simulink model "pfb_fft_pow_ab_16x"  (R2013a) (continued)
== system pfb_fft_pow_ab_16x == (continued)
  pipeline5 : Subsystem  AncestorBlock=casper_library_delays/pipeline  mask(pipeline){latency=1}
      mask help:
      | An explicitly laid-out delay line to avoid mapping to SRL16s
      | for use in pipelining to help achieve timing closure.
      mask init:
      | pipeline_init(gcb,'latency',latency);
      settings: AttributesFormatString=Latency = %<latency>
      mask Display:
      | port_label('input', 1, 'd');
      | port_label('output', 1, 'q');
      | disp(['Z^-', num2str(latency)]);
  pipeline50 : Subsystem  AncestorBlock=casper_library_delays/pipeline  mask(pipeline){latency=1}
      mask help:
      | An explicitly laid-out delay line to avoid mapping to SRL16s
      | for use in pipelining to help achieve timing closure.
      mask init:
      | pipeline_init(gcb,'latency',latency);
      settings: AttributesFormatString=Latency = %<latency>
      mask Display:
      | port_label('input', 1, 'd');
      | port_label('output', 1, 'q');
      | disp(['Z^-', num2str(latency)]);
  pipeline51 : Subsystem  AncestorBlock=casper_library_delays/pipeline  mask(pipeline){latency=1}
      mask help:
      | An explicitly laid-out delay line to avoid mapping to SRL16s
      | for use in pipelining to help achieve timing closure.
      mask init:
      | pipeline_init(gcb,'latency',latency);
      settings: AttributesFormatString=Latency = %<latency>
      mask Display:
      | port_label('input', 1, 'd');
      | port_label('output', 1, 'q');
      | disp(['Z^-', num2str(latency)]);
  pipeline52 : Subsystem  AncestorBlock=casper_library_delays/pipeline  mask(pipeline){latency=2}
      mask help:
      | An explicitly laid-out delay line to avoid mapping to SRL16s
      | for use in pipelining to help achieve timing closure.
      mask init:
      | pipeline_init(gcb,'latency',latency);
      settings: AttributesFormatString=Latency = %<latency>
      mask Display:
      | port_label('input', 1, 'd');
      | port_label('output', 1, 'q');
      | disp(['Z^-', num2str(latency)]);
  pipeline53 : Subsystem  AncestorBlock=casper_library_delays/pipeline  mask(pipeline){latency=1}
      mask help:
      | An explicitly laid-out delay line to avoid mapping to SRL16s
      | for use in pipelining to help achieve timing closure.
      mask init:
      | pipeline_init(gcb,'latency',latency);
      settings: AttributesFormatString=Latency = %<latency>
      mask Display:
      | port_label('input', 1, 'd');
      | port_label('output', 1, 'q');
      | disp(['Z^-', num2str(latency)]);
  pipeline54 : Subsystem  AncestorBlock=casper_library_delays/pipeline  mask(pipeline){latency=2}
      mask help:
      | An explicitly laid-out delay line to avoid mapping to SRL16s
      | for use in pipelining to help achieve timing closure.
      mask init:
      | pipeline_init(gcb,'latency',latency);
      settings: AttributesFormatString=Latency = %<latency>
      mask Display:
      | port_label('input', 1, 'd');
      | port_label('output', 1, 'q');
      | disp(['Z^-', num2str(latency)]);
  pipeline55 : Subsystem  AncestorBlock=casper_library_delays/pipeline  mask(pipeline){latency=2}
      mask help:
      | An explicitly laid-out delay line to avoid mapping to SRL16s
      | for use in pipelining to help achieve timing closure.
      mask init:
      | pipeline_init(gcb,'latency',latency);
      settings: AttributesFormatString=Latency = %<latency>
      mask Display:
      | port_label('input', 1, 'd');
      | port_label('output', 1, 'q');
      | disp(['Z^-', num2str(latency)]);
  pipeline56 : Subsystem  AncestorBlock=casper_library_delays/pipeline  mask(pipeline){latency=2}
      mask help:
      | An explicitly laid-out delay line to avoid mapping to SRL16s
      | for use in pipelining to help achieve timing closure.
      mask init:
      | pipeline_init(gcb,'latency',latency);
      settings: AttributesFormatString=Latency = %<latency>
      mask Display:
      | port_label('input', 1, 'd');
      | port_label('output', 1, 'q');
      | disp(['Z^-', num2str(latency)]);
  pipeline57 : Subsystem  AncestorBlock=casper_library_delays/pipeline  mask(pipeline){latency=2}
      mask help:
      | An explicitly laid-out delay line to avoid mapping to SRL16s
      | for use in pipelining to help achieve timing closure.
      mask init:
      | pipeline_init(gcb,'latency',latency);
      settings: AttributesFormatString=Latency = %<latency>
      mask Display:
      | port_label('input', 1, 'd');
      | port_label('output', 1, 'q');
      | disp(['Z^-', num2str(latency)]);
  pipeline58 : Subsystem  AncestorBlock=casper_library_delays/pipeline  mask(pipeline){latency=1}
      mask help:
      | An explicitly laid-out delay line to avoid mapping to SRL16s
      | for use in pipelining to help achieve timing closure.
      mask init:
      | pipeline_init(gcb,'latency',latency);
      settings: AttributesFormatString=Latency = %<latency>
      mask Display:
      | port_label('input', 1, 'd');
      | port_label('output', 1, 'q');
      | disp(['Z^-', num2str(latency)]);
  pipeline59 : Subsystem  AncestorBlock=casper_library_delays/pipeline  mask(pipeline){latency=1}
      mask help:
      | An explicitly laid-out delay line to avoid mapping to SRL16s
      | for use in pipelining to help achieve timing closure.
      mask init:
      | pipeline_init(gcb,'latency',latency);
      settings: AttributesFormatString=Latency = %<latency>
      mask Display:
      | port_label('input', 1, 'd');
      | port_label('output', 1, 'q');
      | disp(['Z^-', num2str(latency)]);
  pipeline6 : Subsystem  AncestorBlock=casper_library_delays/pipeline  mask(pipeline){latency=1}
      mask help:
      | An explicitly laid-out delay line to avoid mapping to SRL16s
      | for use in pipelining to help achieve timing closure.
      mask init:
      | pipeline_init(gcb,'latency',latency);
      settings: AttributesFormatString=Latency = %<latency>
      mask Display:
      | port_label('input', 1, 'd');
      | port_label('output', 1, 'q');
      | disp(['Z^-', num2str(latency)]);
  pipeline60 : Subsystem  AncestorBlock=casper_library_delays/pipeline  mask(pipeline){latency=1}
      mask help:
      | An explicitly laid-out delay line to avoid mapping to SRL16s
      | for use in pipelining to help achieve timing closure.
      mask init:
      | pipeline_init(gcb,'latency',latency);
      settings: AttributesFormatString=Latency = %<latency>
      mask Display:
      | port_label('input', 1, 'd');
      | port_label('output', 1, 'q');
      | disp(['Z^-', num2str(latency)]);
  pipeline61 : Subsystem  AncestorBlock=casper_library_delays/pipeline  mask(pipeline){latency=1}
      mask help:
      | An explicitly laid-out delay line to avoid mapping to SRL16s
      | for use in pipelining to help achieve timing closure.
      mask init:
      | pipeline_init(gcb,'latency',latency);
      settings: AttributesFormatString=Latency = %<latency>
      mask Display:
      | port_label('input', 1, 'd');
      | port_label('output', 1, 'q');
      | disp(['Z^-', num2str(latency)]);
  pipeline62 : Subsystem  AncestorBlock=casper_library_delays/pipeline  mask(pipeline){latency=1}
      mask help:
      | An explicitly laid-out delay line to avoid mapping to SRL16s
      | for use in pipelining to help achieve timing closure.
      mask init:
      | pipeline_init(gcb,'latency',latency);
      settings: AttributesFormatString=Latency = %<latency>
      mask Display:
      | port_label('input', 1, 'd');
      | port_label('output', 1, 'q');
      | disp(['Z^-', num2str(latency)]);
  pipeline63 : Subsystem  AncestorBlock=casper_library_delays/pipeline  mask(pipeline){latency=1}
      mask help:
      | An explicitly laid-out delay line to avoid mapping to SRL16s
      | for use in pipelining to help achieve timing closure.
      mask init:
      | pipeline_init(gcb,'latency',latency);
      settings: AttributesFormatString=Latency = %<latency>
      mask Display:
      | port_label('input', 1, 'd');
      | port_label('output', 1, 'q');
      | disp(['Z^-', num2str(latency)]);
  pipeline64 : Subsystem  AncestorBlock=casper_library_delays/pipeline  mask(pipeline){latency=1}
      mask help:
      | An explicitly laid-out delay line to avoid mapping to SRL16s
      | for use in pipelining to help achieve timing closure.
      mask init:
      | pipeline_init(gcb,'latency',latency);
      settings: AttributesFormatString=Latency = %<latency>
      mask Display:
      | port_label('input', 1, 'd');
      | port_label('output', 1, 'q');
      | disp(['Z^-', num2str(latency)]);
  pipeline65 : Subsystem  AncestorBlock=casper_library_delays/pipeline  mask(pipeline){latency=1}
      mask help:
      | An explicitly laid-out delay line to avoid mapping to SRL16s
      | for use in pipelining to help achieve timing closure.
      mask init:
      | pipeline_init(gcb,'latency',latency);
      settings: AttributesFormatString=Latency = %<latency>
      mask Display:
      | port_label('input', 1, 'd');
      | port_label('output', 1, 'q');
      | disp(['Z^-', num2str(latency)]);
  pipeline66 : Subsystem  AncestorBlock=casper_library_delays/pipeline  mask(pipeline){latency=1}
      mask help:
      | An explicitly laid-out delay line to avoid mapping to SRL16s
      | for use in pipelining to help achieve timing closure.
      mask init:
      | pipeline_init(gcb,'latency',latency);
      settings: AttributesFormatString=Latency = %<latency>
      mask Display:
      | port_label('input', 1, 'd');
      | port_label('output', 1, 'q');
      | disp(['Z^-', num2str(latency)]);
  pipeline67 : Subsystem  AncestorBlock=casper_library_delays/pipeline  mask(pipeline){latency=1}
      mask help:
      | An explicitly laid-out delay line to avoid mapping to SRL16s
      | for use in pipelining to help achieve timing closure.
      mask init:
      | pipeline_init(gcb,'latency',latency);
      settings: AttributesFormatString=Latency = %<latency>
      mask Display:
      | port_label('input', 1, 'd');
      | port_label('output', 1, 'q');
      | disp(['Z^-', num2str(latency)]);
  pipeline68 : Subsystem  AncestorBlock=casper_library_delays/pipeline  mask(pipeline){latency=1}
      mask help:
      | An explicitly laid-out delay line to avoid mapping to SRL16s
      | for use in pipelining to help achieve timing closure.
      mask init:
      | pipeline_init(gcb,'latency',latency);
      settings: AttributesFormatString=Latency = %<latency>
      mask Display:
      | port_label('input', 1, 'd');
      | port_label('output', 1, 'q');
      | disp(['Z^-', num2str(latency)]);
  pipeline69 : Subsystem  AncestorBlock=casper_library_delays/pipeline  mask(pipeline){latency=1}
      mask help:
      | An explicitly laid-out delay line to avoid mapping to SRL16s
      | for use in pipelining to help achieve timing closure.
      mask init:
      | pipeline_init(gcb,'latency',latency);
      settings: AttributesFormatString=Latency = %<latency>
      mask Display:
      | port_label('input', 1, 'd');
      | port_label('output', 1, 'q');
      | disp(['Z^-', num2str(latency)]);
  pipeline7 : Subsystem  AncestorBlock=casper_library_delays/pipeline  mask(pipeline){latency=1}
      mask help:
      | An explicitly laid-out delay line to avoid mapping to SRL16s
      | for use in pipelining to help achieve timing closure.
      mask init:
      | pipeline_init(gcb,'latency',latency);
      settings: AttributesFormatString=Latency = %<latency>
      mask Display:
      | port_label('input', 1, 'd');
      | port_label('output', 1, 'q');
      | disp(['Z^-', num2str(latency)]);
  pipeline70 : Subsystem  AncestorBlock=casper_library_delays/pipeline  mask(pipeline){latency=1}
      mask help:
      | An explicitly laid-out delay line to avoid mapping to SRL16s
      | for use in pipelining to help achieve timing closure.
      mask init:
      | pipeline_init(gcb,'latency',latency);
      settings: AttributesFormatString=Latency = %<latency>
      mask Display:
      | port_label('input', 1, 'd');
      | port_label('output', 1, 'q');
      | disp(['Z^-', num2str(latency)]);
  pipeline71 : Subsystem  AncestorBlock=casper_library_delays/pipeline  mask(pipeline){latency=1}
      mask help:
      | An explicitly laid-out delay line to avoid mapping to SRL16s
      | for use in pipelining to help achieve timing closure.
      mask init:
      | pipeline_init(gcb,'latency',latency);
      settings: AttributesFormatString=Latency = %<latency>
      mask Display:
      | port_label('input', 1, 'd');
      | port_label('output', 1, 'q');
      | disp(['Z^-', num2str(latency)]);
  pipeline72 : Subsystem  AncestorBlock=casper_library_delays/pipeline  mask(pipeline){latency=1}
      mask help:
      | An explicitly laid-out delay line to avoid mapping to SRL16s
      | for use in pipelining to help achieve timing closure.
      mask init:
      | pipeline_init(gcb,'latency',latency);
      settings: AttributesFormatString=Latency = %<latency>
      mask Display:
      | port_label('input', 1, 'd');
      | port_label('output', 1, 'q');
      | disp(['Z^-', num2str(latency)]);
  pipeline73 : Subsystem  AncestorBlock=casper_library_delays/pipeline  mask(pipeline){latency=1}
      mask help:
      | An explicitly laid-out delay line to avoid mapping to SRL16s
      | for use in pipelining to help achieve timing closure.
      mask init:
      | pipeline_init(gcb,'latency',latency);
      settings: AttributesFormatString=Latency = %<latency>
      mask Display:
      | port_label('input', 1, 'd');
      | port_label('output', 1, 'q');
      | disp(['Z^-', num2str(latency)]);
  pipeline74 : Subsystem  AncestorBlock=casper_library_delays/pipeline  mask(pipeline){latency=1}
      mask help:
      | An explicitly laid-out delay line to avoid mapping to SRL16s
      | for use in pipelining to help achieve timing closure.
      mask init:
      | pipeline_init(gcb,'latency',latency);
      settings: AttributesFormatString=Latency = %<latency>
      mask Display:
      | port_label('input', 1, 'd');
      | port_label('output', 1, 'q');
      | disp(['Z^-', num2str(latency)]);
  pipeline75 : Subsystem  AncestorBlock=casper_library_delays/pipeline  mask(pipeline){latency=1}
      mask help:
      | An explicitly laid-out delay line to avoid mapping to SRL16s
      | for use in pipelining to help achieve timing closure.
      mask init:
      | pipeline_init(gcb,'latency',latency);
      settings: AttributesFormatString=Latency = %<latency>
      mask Display:
      | port_label('input', 1, 'd');
      | port_label('output', 1, 'q');
      | disp(['Z^-', num2str(latency)]);
  pipeline76 : Subsystem  AncestorBlock=casper_library_delays/pipeline  mask(pipeline){latency=1}
      mask help:
      | An explicitly laid-out delay line to avoid mapping to SRL16s
      | for use in pipelining to help achieve timing closure.
      mask init:
      | pipeline_init(gcb,'latency',latency);
      settings: AttributesFormatString=Latency = %<latency>
      mask Display:
      | port_label('input', 1, 'd');
      | port_label('output', 1, 'q');
      | disp(['Z^-', num2str(latency)]);
  pipeline77 : Subsystem  AncestorBlock=casper_library_delays/pipeline  mask(pipeline){latency=1}
      mask help:
      | An explicitly laid-out delay line to avoid mapping to SRL16s
      | for use in pipelining to help achieve timing closure.
      mask init:
      | pipeline_init(gcb,'latency',latency);
      settings: AttributesFormatString=Latency = %<latency>
      mask Display:
      | port_label('input', 1, 'd');
      | port_label('output', 1, 'q');
      | disp(['Z^-', num2str(latency)]);
  pipeline78 : Subsystem  AncestorBlock=casper_library_delays/pipeline  mask(pipeline){latency=1}
      mask help:
      | An explicitly laid-out delay line to avoid mapping to SRL16s
      | for use in pipelining to help achieve timing closure.
      mask init:
      | pipeline_init(gcb,'latency',latency);
      settings: AttributesFormatString=Latency = %<latency>
      mask Display:
      | port_label('input', 1, 'd');
      | port_label('output', 1, 'q');
      | disp(['Z^-', num2str(latency)]);
  pipeline79 : Subsystem  AncestorBlock=casper_library_delays/pipeline  mask(pipeline){latency=1}
      mask help:
      | An explicitly laid-out delay line to avoid mapping to SRL16s
      | for use in pipelining to help achieve timing closure.
      mask init:
      | pipeline_init(gcb,'latency',latency);
      settings: AttributesFormatString=Latency = %<latency>
      mask Display:
      | port_label('input', 1, 'd');
      | port_label('output', 1, 'q');
      | disp(['Z^-', num2str(latency)]);
  pipeline8 : Subsystem  AncestorBlock=casper_library_delays/pipeline  mask(pipeline){latency=1}
      mask help:
      | An explicitly laid-out delay line to avoid mapping to SRL16s
      | for use in pipelining to help achieve timing closure.
      mask init:
      | pipeline_init(gcb,'latency',latency);
      settings: AttributesFormatString=Latency = %<latency>
      mask Display:
      | port_label('input', 1, 'd');
      | port_label('output', 1, 'q');
      | disp(['Z^-', num2str(latency)]);
  pipeline80 : Subsystem  AncestorBlock=casper_library_delays/pipeline  mask(pipeline){latency=1}
      mask help:
      | An explicitly laid-out delay line to avoid mapping to SRL16s
      | for use in pipelining to help achieve timing closure.
      mask init:
      | pipeline_init(gcb,'latency',latency);
      settings: AttributesFormatString=Latency = %<latency>
      mask Display:
      | port_label('input', 1, 'd');
      | port_label('output', 1, 'q');
      | disp(['Z^-', num2str(latency)]);
  pipeline81 : Subsystem  AncestorBlock=casper_library_delays/pipeline  mask(pipeline){latency=1}
      mask help:
      | An explicitly laid-out delay line to avoid mapping to SRL16s
      | for use in pipelining to help achieve timing closure.
      mask init:
      | pipeline_init(gcb,'latency',latency);
      settings: AttributesFormatString=Latency = %<latency>
      mask Display:
      | port_label('input', 1, 'd');
      | port_label('output', 1, 'q');
      | disp(['Z^-', num2str(latency)]);
  pipeline82 : Subsystem  AncestorBlock=casper_library_delays/pipeline  mask(pipeline){latency=1}
      mask help:
      | An explicitly laid-out delay line to avoid mapping to SRL16s
      | for use in pipelining to help achieve timing closure.
      mask init:
      | pipeline_init(gcb,'latency',latency);
      settings: AttributesFormatString=Latency = %<latency>
      mask Display:
      | port_label('input', 1, 'd');
      | port_label('output', 1, 'q');
      | disp(['Z^-', num2str(latency)]);
  pipeline83 : Subsystem  AncestorBlock=casper_library_delays/pipeline  mask(pipeline){latency=1}
      mask help:
      | An explicitly laid-out delay line to avoid mapping to SRL16s
      | for use in pipelining to help achieve timing closure.
      mask init:
      | pipeline_init(gcb,'latency',latency);
      settings: AttributesFormatString=Latency = %<latency>
      mask Display:
      | port_label('input', 1, 'd');
      | port_label('output', 1, 'q');
      | disp(['Z^-', num2str(latency)]);
  pipeline84 : Subsystem  AncestorBlock=casper_library_delays/pipeline  mask(pipeline){latency=2}
      mask help:
      | An explicitly laid-out delay line to avoid mapping to SRL16s
      | for use in pipelining to help achieve timing closure.
      mask init:
      | pipeline_init(gcb,'latency',latency);
      settings: AttributesFormatString=Latency = %<latency>
      mask Display:
      | port_label('input', 1, 'd');
      | port_label('output', 1, 'q');
      | disp(['Z^-', num2str(latency)]);
  pipeline85 : Subsystem  AncestorBlock=casper_library_delays/pipeline  mask(pipeline){latency=1}
      mask help:
      | An explicitly laid-out delay line to avoid mapping to SRL16s
      | for use in pipelining to help achieve timing closure.
      mask init:
      | pipeline_init(gcb,'latency',latency);
      settings: AttributesFormatString=Latency = %<latency>
      mask Display:
      | port_label('input', 1, 'd');
      | port_label('output', 1, 'q');
      | disp(['Z^-', num2str(latency)]);
  pipeline86 : Subsystem  AncestorBlock=casper_library_delays/pipeline  mask(pipeline){latency=2}
      mask help:
      | An explicitly laid-out delay line to avoid mapping to SRL16s
      | for use in pipelining to help achieve timing closure.
      mask init:
      | pipeline_init(gcb,'latency',latency);
      settings: AttributesFormatString=Latency = %<latency>
      mask Display:
      | port_label('input', 1, 'd');
      | port_label('output', 1, 'q');
      | disp(['Z^-', num2str(latency)]);
  pipeline87 : Subsystem  AncestorBlock=casper_library_delays/pipeline  mask(pipeline){latency=2}
      mask help:
      | An explicitly laid-out delay line to avoid mapping to SRL16s
      | for use in pipelining to help achieve timing closure.
      mask init:
      | pipeline_init(gcb,'latency',latency);
      settings: AttributesFormatString=Latency = %<latency>
      mask Display:
      | port_label('input', 1, 'd');
      | port_label('output', 1, 'q');
      | disp(['Z^-', num2str(latency)]);
  pipeline88 : Subsystem  AncestorBlock=casper_library_delays/pipeline  mask(pipeline){latency=2}
      mask help:
      | An explicitly laid-out delay line to avoid mapping to SRL16s
      | for use in pipelining to help achieve timing closure.
      mask init:
      | pipeline_init(gcb,'latency',latency);
      settings: AttributesFormatString=Latency = %<latency>
      mask Display:
      | port_label('input', 1, 'd');
      | port_label('output', 1, 'q');
      | disp(['Z^-', num2str(latency)]);
  pipeline89 : Subsystem  AncestorBlock=casper_library_delays/pipeline  mask(pipeline){latency=2}
      mask help:
      | An explicitly laid-out delay line to avoid mapping to SRL16s
      | for use in pipelining to help achieve timing closure.
      mask init:
      | pipeline_init(gcb,'latency',latency);
      settings: AttributesFormatString=Latency = %<latency>
      mask Display:
      | port_label('input', 1, 'd');
      | port_label('output', 1, 'q');
      | disp(['Z^-', num2str(latency)]);
  pipeline9 : Subsystem  AncestorBlock=casper_library_delays/pipeline  mask(pipeline){latency=1}
      mask help:
      | An explicitly laid-out delay line to avoid mapping to SRL16s
      | for use in pipelining to help achieve timing closure.
      mask init:
      | pipeline_init(gcb,'latency',latency);
      settings: AttributesFormatString=Latency = %<latency>
      mask Display:
      | port_label('input', 1, 'd');
      | port_label('output', 1, 'q');
      | disp(['Z^-', num2str(latency)]);
  pipeline90 : Subsystem  AncestorBlock=casper_library_delays/pipeline  mask(pipeline){latency=1}
      mask help:
      | An explicitly laid-out delay line to avoid mapping to SRL16s
      | for use in pipelining to help achieve timing closure.
      mask init:
      | pipeline_init(gcb,'latency',latency);
      settings: AttributesFormatString=Latency = %<latency>
      mask Display:
      | port_label('input', 1, 'd');
      | port_label('output', 1, 'q');
      | disp(['Z^-', num2str(latency)]);
  pipeline91 : Subsystem  AncestorBlock=casper_library_delays/pipeline  mask(pipeline){latency=1}
      mask help:
      | An explicitly laid-out delay line to avoid mapping to SRL16s
      | for use in pipelining to help achieve timing closure.
      mask init:
      | pipeline_init(gcb,'latency',latency);
      settings: AttributesFormatString=Latency = %<latency>
      mask Display:
      | port_label('input', 1, 'd');
      | port_label('output', 1, 'q');
      | disp(['Z^-', num2str(latency)]);
  pipeline92 : Subsystem  AncestorBlock=casper_library_delays/pipeline  mask(pipeline){latency=1}
      mask help:
      | An explicitly laid-out delay line to avoid mapping to SRL16s
      | for use in pipelining to help achieve timing closure.
      mask init:
      | pipeline_init(gcb,'latency',latency);
      settings: AttributesFormatString=Latency = %<latency>
      mask Display:
      | port_label('input', 1, 'd');
      | port_label('output', 1, 'q');
      | disp(['Z^-', num2str(latency)]);
  pipeline93 : Subsystem  AncestorBlock=casper_library_delays/pipeline  mask(pipeline){latency=1}
      mask help:
      | An explicitly laid-out delay line to avoid mapping to SRL16s
      | for use in pipelining to help achieve timing closure.
      mask init:
      | pipeline_init(gcb,'latency',latency);
      settings: AttributesFormatString=Latency = %<latency>
      mask Display:
      | port_label('input', 1, 'd');
      | port_label('output', 1, 'q');
      | disp(['Z^-', num2str(latency)]);
  pipeline94 : Subsystem  AncestorBlock=casper_library_delays/pipeline  mask(pipeline){latency=1}
      mask help:
      | An explicitly laid-out delay line to avoid mapping to SRL16s
      | for use in pipelining to help achieve timing closure.
      mask init:
      | pipeline_init(gcb,'latency',latency);
      settings: AttributesFormatString=Latency = %<latency>
      mask Display:
      | port_label('input', 1, 'd');
      | port_label('output', 1, 'q');
      | disp(['Z^-', num2str(latency)]);
  pipeline95 : Subsystem  AncestorBlock=casper_library_delays/pipeline  mask(pipeline){latency=1}
      mask help:
      | An explicitly laid-out delay line to avoid mapping to SRL16s
      | for use in pipelining to help achieve timing closure.
      mask init:
      | pipeline_init(gcb,'latency',latency);
      settings: AttributesFormatString=Latency = %<latency>
      mask Display:
      | port_label('input', 1, 'd');
      | port_label('output', 1, 'q');
      | disp(['Z^-', num2str(latency)]);
  pipeline96 : Subsystem  AncestorBlock=casper_library_delays/pipeline  mask(pipeline){latency=1}
      mask help:
      | An explicitly laid-out delay line to avoid mapping to SRL16s
      | for use in pipelining to help achieve timing closure.
      mask init:
      | pipeline_init(gcb,'latency',latency);
      settings: AttributesFormatString=Latency = %<latency>
      mask Display:
      | port_label('input', 1, 'd');
      | port_label('output', 1, 'q');
      | disp(['Z^-', num2str(latency)]);
  pipeline97 : Subsystem  AncestorBlock=casper_library_delays/pipeline  mask(pipeline){latency=1}
      mask help:
      | An explicitly laid-out delay line to avoid mapping to SRL16s
      | for use in pipelining to help achieve timing closure.
      mask init:
      | pipeline_init(gcb,'latency',latency);
      settings: AttributesFormatString=Latency = %<latency>
      mask Display:
      | port_label('input', 1, 'd');
      | port_label('output', 1, 'q');
      | disp(['Z^-', num2str(latency)]);
  pipeline98 : Subsystem  AncestorBlock=casper_library_delays/pipeline  mask(pipeline){latency=1}
      mask help:
      | An explicitly laid-out delay line to avoid mapping to SRL16s
      | for use in pipelining to help achieve timing closure.
      mask init:
      | pipeline_init(gcb,'latency',latency);
      settings: AttributesFormatString=Latency = %<latency>
      mask Display:
      | port_label('input', 1, 'd');
      | port_label('output', 1, 'q');
      | disp(['Z^-', num2str(latency)]);
  pipeline99 : Subsystem  AncestorBlock=casper_library_delays/pipeline  mask(pipeline){latency=1}
      mask help:
      | An explicitly laid-out delay line to avoid mapping to SRL16s
      | for use in pipelining to help achieve timing closure.
      mask init:
      | pipeline_init(gcb,'latency',latency);
      settings: AttributesFormatString=Latency = %<latency>
      mask Display:
      | port_label('input', 1, 'd');
      | port_label('output', 1, 'q');
      | disp(['Z^-', num2str(latency)]);
  pow_quant_0 : Subsystem
  pow_quant_1 : Subsystem
  pow_quant_2 : Subsystem
  pow_quant_3 : Subsystem
  power_integrator_16x : Subsystem
  ri_to_c : casper_library_misc/ri_to_c
  snap_adc : Subsystem
  sync_gen : Subsystem  AncestorBlock=casper_library_misc/sync_gen  mask(sync_gen){sim_acc_len=1, fft_size=256, fft_simult_inputs=1, pfb_fir_taps=4, reorder_vec=[2 2 2], scale=1}
      mask prompts: sim_acc_len "Simulation Accumulation Length"; fft_size "FFT Size"; fft_simult_inputs "Simultaneous Inputs (FFT)"; pfb_fir_taps "Taps in pfb_fir (use 1 if no pfb_fir)"; reorder_vec "Reorder Orders (e.g. [2 2 2])"
      mask help:
      | This block computes the sync period based on system parameters as
      | described in Casper memo.  Detailed descritions of the block's
      | variables can be found in the 'Block Documentation' section of
      | the CASPER wiki.
      mask init:
      | sync_gen_init(gcb, ...
      |     'sim_acc_len', sim_acc_len, ...
      |     'fft_size', fft_size, ...
      |     'fft_simult_inputs', fft_simult_inputs,...
      |     'pfb_fir_taps', pfb_fir_taps, ...
      |     'reorder_vec', reorder_vec, ...
      |     'scale', scale);
      settings: AttributesFormatString=sim_sync_period=2048
      mask Help:
      | SyncPeriod=LCM(reorder_vec)*(acc_num)*(pfb_fir_len)*(fft_size/fft_simult_inputs)
      | 
      | See CASPER sync pulse memo for more details.
  xab0 : Subsystem
  xab1 : Subsystem
  xab2 : Subsystem
  xab3 : Subsystem
  xpow0 : Subsystem
  xpow1 : Subsystem
  xpow2 : Subsystem
  xpow3 : Subsystem
  tag scope: hold_data=global
  wires:
  adc16x250-8/3(a3) -> Bus Creator/3
  adc16x250-8/2(a2) -> Bus Creator/2
  adc16x250-8/4(a4) -> Bus Creator/4
  adc16x250-8/1(a1) -> Bus Creator/1
  Bus Creator/1 -> [adc16_chan_a]
  adc16x250-8/8(b4) -> Bus Creator1/4
  adc16x250-8/6(b2) -> Bus Creator1/2
  adc16x250-8/7(b3) -> Bus Creator1/3
  adc16x250-8/5(b1) -> Bus Creator1/1
  adc16x250-8/12(c4) -> Bus Creator2/4
  adc16x250-8/11(c3) -> Bus Creator2/3
  adc16x250-8/10(c2) -> Bus Creator2/2
  adc16x250-8/9(c1) -> Bus Creator2/1
  adc16x250-8/15(d3) -> Bus Creator3/3
  adc16x250-8/16(d4) -> Bus Creator3/4
  adc16x250-8/14(d2) -> Bus Creator3/2
  adc16x250-8/13(d1) -> Bus Creator3/1
  Bus Creator1/1 -> [adc16_chan_b]
  Bus Creator2/1 -> [adc16_chan_c]
  Bus Creator3/1 -> [adc16_chan_d]
  Gain/1 -> adc16x250-8/1(a1_sim)
  Gain1/1 -> adc16x250-8/2(a2_sim)
  Gain2/1 -> adc16x250-8/3(a3_sim)
  Gain3/1 -> adc16x250-8/4(a4_sim)
  Gain4/1 -> adc16x250-8/5(b1_sim)
  Gain5/1 -> adc16x250-8/6(b2_sim)
  Gain6/1 -> adc16x250-8/7(b3_sim)
  Gain7/1 -> adc16x250-8/8(b4_sim)
  Gain10/1 -> adc16x250-8/11(c3_sim)
  Gain8/1 -> adc16x250-8/9(c1_sim)
  Gain12/1 -> adc16x250-8/13(d1_sim)
  Gain15/1 -> adc16x250-8/16(d4_sim)
  Gain11/1 -> adc16x250-8/12(c4_sim)
  Gain14/1 -> adc16x250-8/15(d3_sim)
  Gain9/1 -> adc16x250-8/10(c2_sim)
  Gain13/1 -> adc16x250-8/14(d2_sim)
  [adc16_chan_a] -> Bus Selector/1
  generators/1(Out1) -> Gain/1
  generators/2(Out2) -> Gain1/1
  generators/3(Out3) -> Gain2/1
  generators/4(Out4) -> Gain3/1
  generators/5(Out5) -> Gain4/1
  generators/6(Out6) -> Gain5/1
  generators/7(Out7) -> Gain6/1
  generators/8(Out8) -> Gain7/1
  generators/9(Out9) -> Gain8/1
  generators/10(Out10) -> Gain9/1
  generators/11(Out11) -> Gain10/1
  generators/12(Out12) -> Gain11/1
  generators/13(Out13) -> Gain12/1
  generators/14(Out14) -> Gain13/1
  generators/15(Out15) -> Gain14/1
  generators/16(Out16) -> Gain15/1
  sync_gen/1(sync_out) -> pipeline34/1(d)
  pipeline34/1(q) -> [sync_gen]
  Bus Selector/1 -> pipeline24/1(d)  "<a1>"
  Bus Selector/2 -> pipeline26/1(d)  "<a2>"
  Bus Selector/3 -> pipeline27/1(d)  "<a3>"
  Bus Selector/4 -> pipeline28/1(d)  "<a4>"
  [sync_gen] -> pipeline29/1(d)
  [sync_mul_quant] -> acc_cntrl/2(sync)
  [cal_acc_len] -> acc_cntrl/1(acc_len)
  [cnt_rst] -> acc_cntrl/3(rst)
  acc_cntrl/1(new_acc) -> [cal_new_acc]
  edge_detect/1(out) -> Counter1/2
  Counter1/1 -> cal_acc_count/1(out_reg)
  Counter/1 -> [cal_addr]
  edge_detect1/1(out) -> Counter/1
  pipeline50/1(q) -> Counter/2
  pipeline214/1(q) -> [cal_we]
  acc_control/1(cal_we) -> edge_detect/1(in), edge_detect1/1(in), pipeline50/1(d), pipeline214/1(d)
  call_new_acc/1(in_reg) -> edge_detect3/1(in)
  edge_detect3/1(out) -> acc_control/1(call_new_acc)
  cal_acc_len/1(in_reg) -> [cal_acc_len]
  gain/1(in_reg) -> [gain]
  [cal_we] -> xpow0/1(we)
  [cal_addr] -> xpow0/2(addr)
  [cal_new_acc] -> xpow0/3(new_acc)
  xpow0/1(valid) -> [valid]
  [valid] -> acc_control/2(valid)
  [sync_fft] -> pow_quant_0/1(sync)
  [a1] -> pow_quant_0/2(in0)
  [a2] -> pow_quant_0/3(in1)
  [a3] -> pow_quant_0/4(in2)
  [a4] -> pow_quant_0/5(in3)
  [gain] -> pow_quant_0/6(gain)
  pow_quant_0/1(sync_out) -> pipeline6/1(d)
  pow_quant_0/2(out0) -> pipeline5/1(d)
  pow_quant_0/3(out1) -> pipeline4/1(d)
  pow_quant_0/4(out2) -> pipeline1/1(d)
  pow_quant_0/5(out3) -> pipeline3/1(d)
  pipeline7/1(q) -> Terminator11/1
  [cal_we] -> xab0/1(we)
  [cal_addr] -> xab0/2(addr)
  [cal_new_acc] -> xab0/3(new_acc)
  xab0/1(valid) -> Terminator2/1
  [sync_fft] -> mul_quant_0/1(sync)
  [gain] -> mul_quant_0/7(gain)
  [a1] -> mul_quant_0/2(s_ref)
  [a1] -> mul_quant_0/3(s0)
  [a2] -> mul_quant_0/4(s1)
  [a3] -> mul_quant_0/5(s2)
  [a4] -> mul_quant_0/6(s3)
  mul_quant_0/1(sync_out) -> pipeline7/1(d)
  mul_quant_0/2(ab_re0) -> pipeline8/1(d)
  mul_quant_0/3(ab_im0) -> pipeline9/1(d)
  mul_quant_0/4(ab_re1) -> pipeline10/1(d)
  mul_quant_0/5(ab_im1) -> pipeline11/1(d)
  mul_quant_0/6(ab_re2) -> pipeline14/1(d)
  mul_quant_0/7(ab_im2) -> pipeline15/1(d)
  mul_quant_0/8(ab_re3) -> pipeline12/1(d)
  mul_quant_0/9(ab_im3) -> pipeline13/1(d)
  pfb_fft_0/1(sync_out) -> pipeline33/1(d)
  pfb_fft_0/2(pol0_out) -> pipeline32/1(d)
  pfb_fft_0/3(pol1_out) -> pipeline31/1(d)
  pfb_fft_0/4(pol2_out) -> pipeline30/1(d)
  pfb_fft_0/5(pol3_out) -> pipeline25/1(d)
  cnt_rst/1(in_reg) -> [cnt_rst]
  Constant2/1 -> snap_adc/5(trig)
  [adc16_chan_c] -> snap_adc/3(data_c)
  [adc16_chan_b] -> snap_adc/2(data_b)
  [adc16_chan_d] -> snap_adc/4(data_d)
  [adc16_chan_a] -> snap_adc/1(data_a)
  Constant3/1 -> power_integrator_16x/5(acc_len)
  [adc16_chan_a] -> power_integrator_16x/1(chan_a)
  [adc16_chan_b] -> power_integrator_16x/2(chan_b)
  [adc16_chan_c] -> power_integrator_16x/3(chan_c)
  [adc16_chan_d] -> power_integrator_16x/4(chan_d)
  [cnt_rst] -> Counter1/1
  phase_cal_re/1(in_reg) -> Reinterpret/1
  phase_cal_im/1(in_reg) -> Reinterpret17/1
  Reinterpret/1 -> Convert/1
  Reinterpret17/1 -> Convert1/1
  Convert/1 -> ri_to_c/1
  Convert1/1 -> ri_to_c/2
  ri_to_c/1 -> [phase_val]
  phase_0/1(sync_out) -> [sync_fft]
  phase_0/3(out2) -> [a2]
  phase_0/4(out3) -> [a3]
  phase_0/5(out4) -> [a4]
  phase_0/2(out1) -> [a1]
  [phase_cal_a1] -> phase_0/6(phase1)
  [phase_cal_a2] -> phase_0/7(phase2)
  [phase_cal_a3] -> phase_0/8(phase3)
  [phase_cal_a4] -> phase_0/9(phase4)
  pipeline6/1(q) -> [sync_mul_quant]
  pipeline1/1(q) -> xpow0/6(din3)
  pipeline5/1(q) -> xpow0/4(din1)
  pipeline3/1(q) -> xpow0/7(din4)
  pipeline4/1(q) -> xpow0/5(din2)
  pipeline23/1(q) -> xab0/5(im0)
  pipeline18/1(q) -> xab0/10(re3)
  pipeline19/1(q) -> xab0/11(im3)
  pipeline20/1(q) -> xab0/8(re2)
  pipeline22/1(q) -> xab0/4(re0)
  pipeline21/1(q) -> xab0/9(im2)
  pipeline17/1(q) -> xab0/7(im1)
  pipeline16/1(q) -> xab0/6(re1)
  pipeline8/1(q) -> pipeline22/1(d)
  pipeline9/1(q) -> pipeline23/1(d)
  pipeline10/1(q) -> pipeline16/1(d)
  pipeline11/1(q) -> pipeline17/1(d)
  pipeline14/1(q) -> pipeline20/1(d)
  pipeline15/1(q) -> pipeline21/1(d)
  pipeline12/1(q) -> pipeline18/1(d)
  pipeline13/1(q) -> pipeline19/1(d)
  pipeline28/1(q) -> pfb_fft_0/5(pol3_in)
  pipeline27/1(q) -> pfb_fft_0/4(pol2_in)
  pipeline24/1(q) -> pfb_fft_0/2(pol0_in)
  pipeline26/1(q) -> pfb_fft_0/3(pol1_on)
  pipeline29/1(q) -> pfb_fft_0/1(sync)
  pipeline33/1(q) -> phase_0/1(sync)
  pipeline32/1(q) -> [fft_a1]
  pipeline31/1(q) -> [fft_a2]
  pipeline30/1(q) -> [fft_a3]
  pipeline25/1(q) -> [fft_a4]
  [fft_a1] -> phase_0/2(in1)
  [fft_a2] -> phase_0/3(in2)
  [fft_a3] -> phase_0/4(in3)
  [fft_a4] -> phase_0/5(in4)
  Slice2/1 -> edge_detect4/1
  pipeline35/1 -> Slice2/1
  hold_data/1(in_reg) -> pipeline35/1
  edge_detect4/1 -> [hold_data]
  phase_cal_we/1(in_reg) -> edge_detect2/1
  enable0/1(phase_out) -> [phase_cal_a1]
  [phase_val] -> enable0/1(phase_in)
  [phase_we] -> enable0/3(we)
  [phase_addr] -> enable0/2(addr)
  phase_cal_addr/1(in_reg) -> pipeline/1(d)
  edge_detect2/1 -> pipeline2/1(d)
  pipeline/1(q) -> [phase_addr]
  pipeline2/1(q) -> [phase_we]
  enable1/1(phase_out) -> [phase_cal_a2]
  [phase_val] -> enable1/1(phase_in)
  [phase_we] -> enable1/3(we)
  [phase_addr] -> enable1/2(addr)
  enable2/1(phase_out) -> [phase_cal_a3]
  [phase_val] -> enable2/1(phase_in)
  [phase_we] -> enable2/3(we)
  [phase_addr] -> enable2/2(addr)
  enable3/1(phase_out) -> [phase_cal_a4]
  [phase_val] -> enable3/1(phase_in)
  [phase_we] -> enable3/3(we)
  [phase_addr] -> enable3/2(addr)
  [hold_data] -> power_integrator_16x/6(trig)
  enable4/1(phase_out) -> [phase_cal_b1]
  [phase_val] -> enable4/1(phase_in)
  [phase_we] -> enable4/3(we)
  [phase_addr] -> enable4/2(addr)
  enable5/1(phase_out) -> [phase_cal_b2]
  [phase_val] -> enable5/1(phase_in)
  [phase_we] -> enable5/3(we)
  [phase_addr] -> enable5/2(addr)
  enable6/1(phase_out) -> [phase_cal_b3]
  [phase_val] -> enable6/1(phase_in)
  [phase_we] -> enable6/3(we)
  [phase_addr] -> enable6/2(addr)
  enable7/1(phase_out) -> [phase_cal_b4]
  [phase_val] -> enable7/1(phase_in)
  [phase_we] -> enable7/3(we)
  [phase_addr] -> enable7/2(addr)
  enable8/1(phase_out) -> [phase_cal_c1]
  [phase_val] -> enable8/1(phase_in)
  [phase_we] -> enable8/3(we)
  [phase_addr] -> enable8/2(addr)
  enable9/1(phase_out) -> [phase_cal_c2]
  [phase_val] -> enable9/1(phase_in)
  [phase_we] -> enable9/3(we)
  [phase_addr] -> enable9/2(addr)
  enable10/1(phase_out) -> [phase_cal_c3]
  [phase_val] -> enable10/1(phase_in)
  [phase_we] -> enable10/3(we)
  [phase_addr] -> enable10/2(addr)
  enable11/1(phase_out) -> [phase_cal_c4]
  [phase_val] -> enable11/1(phase_in)
  [phase_we] -> enable11/3(we)
  [phase_addr] -> enable11/2(addr)
  enable14/1(phase_out) -> [phase_cal_d3]
  [phase_val] -> enable14/1(phase_in)
  [phase_we] -> enable14/3(we)
  [phase_addr] -> enable14/2(addr)
  enable15/1(phase_out) -> [phase_cal_d4]
  [phase_val] -> enable15/1(phase_in)
  [phase_we] -> enable15/3(we)
  [phase_addr] -> enable15/2(addr)
  enable12/1(phase_out) -> [phase_cal_d1]
  [phase_val] -> enable12/1(phase_in)
  [phase_we] -> enable12/3(we)
  [phase_addr] -> enable12/2(addr)
  enable13/1(phase_out) -> [phase_cal_d2]
  [phase_val] -> enable13/1(phase_in)
  [phase_we] -> enable13/3(we)
  [phase_addr] -> enable13/2(addr)
  [adc16_chan_b] -> Bus Selector1/1
  Bus Selector1/1 -> pipeline52/1(d)  "<b1>"
  Bus Selector1/2 -> pipeline54/1(d)  "<b2>"
  Bus Selector1/3 -> pipeline55/1(d)  "<b3>"
  Bus Selector1/4 -> pipeline56/1(d)  "<b4>"
  [sync_gen] -> pipeline57/1(d)
  [cal_we] -> xpow1/1(we)
  [cal_addr] -> xpow1/2(addr)
  [cal_new_acc] -> xpow1/3(new_acc)
  xpow1/1(valid) -> Terminator6/1
  [sync_fft] -> pow_quant_1/1(sync)
  [b1] -> pow_quant_1/2(in0)
  [b2] -> pow_quant_1/3(in1)
  [b3] -> pow_quant_1/4(in2)
  [b4] -> pow_quant_1/5(in3)
  [gain] -> pow_quant_1/6(gain)
  pow_quant_1/1(sync_out) -> pipeline65/1(d)
  pow_quant_1/2(out0) -> pipeline64/1(d)
  pow_quant_1/3(out1) -> pipeline63/1(d)
  pow_quant_1/4(out2) -> pipeline36/1(d)
  pow_quant_1/5(out3) -> pipeline58/1(d)
  pipeline66/1(q) -> Terminator1/1
  [cal_we] -> xab1/1(we)
  [cal_addr] -> xab1/2(addr)
  [cal_new_acc] -> xab1/3(new_acc)
  xab1/1(valid) -> Terminator3/1
  [sync_fft] -> mul_quant_1/1(sync)
  [gain] -> mul_quant_1/7(gain)
  [a1] -> mul_quant_1/2(s_ref)
  [b1] -> mul_quant_1/3(s0)
  [b2] -> mul_quant_1/4(s1)
  [b3] -> mul_quant_1/5(s2)
  [b4] -> mul_quant_1/6(s3)
  mul_quant_1/1(sync_out) -> pipeline66/1(d)
  mul_quant_1/2(ab_re0) -> pipeline67/1(d)
  mul_quant_1/3(ab_im0) -> pipeline68/1(d)
  mul_quant_1/4(ab_re1) -> pipeline37/1(d)
  mul_quant_1/5(ab_im1) -> pipeline38/1(d)
  mul_quant_1/6(ab_re2) -> pipeline41/1(d)
  mul_quant_1/7(ab_im2) -> pipeline42/1(d)
  mul_quant_1/8(ab_re3) -> pipeline39/1(d)
  mul_quant_1/9(ab_im3) -> pipeline40/1(d)
  pfb_fft_1/1(sync_out) -> pipeline62/1(d)
  pfb_fft_1/2(pol0_out) -> pipeline61/1(d)
  pfb_fft_1/3(pol1_out) -> pipeline60/1(d)
  pfb_fft_1/4(pol2_out) -> pipeline59/1(d)
  pfb_fft_1/5(pol3_out) -> pipeline53/1(d)
  phase_1/1(sync_out) -> Terminator5/1
  phase_1/3(out2) -> [b2]
  phase_1/4(out3) -> [b3]
  phase_1/5(out4) -> [b4]
  phase_1/2(out1) -> [b1]
  [phase_cal_b1] -> phase_1/6(phase1)
  [phase_cal_b2] -> phase_1/7(phase2)
  [phase_cal_b3] -> phase_1/8(phase3)
  [phase_cal_b4] -> phase_1/9(phase4)
  pipeline65/1(q) -> Terminator4/1
  pipeline36/1(q) -> xpow1/6(din3)
  pipeline64/1(q) -> xpow1/4(din1)
  pipeline58/1(q) -> xpow1/7(din4)
  pipeline63/1(q) -> xpow1/5(din2)
  pipeline51/1(q) -> xab1/5(im0)
  pipeline45/1(q) -> xab1/10(re3)
  pipeline46/1(q) -> xab1/11(im3)
  pipeline47/1(q) -> xab1/8(re2)
  pipeline49/1(q) -> xab1/4(re0)
  pipeline48/1(q) -> xab1/9(im2)
  pipeline44/1(q) -> xab1/7(im1)
  pipeline43/1(q) -> xab1/6(re1)
  pipeline67/1(q) -> pipeline49/1(d)
  pipeline68/1(q) -> pipeline51/1(d)
  pipeline37/1(q) -> pipeline43/1(d)
  pipeline38/1(q) -> pipeline44/1(d)
  pipeline41/1(q) -> pipeline47/1(d)
  pipeline42/1(q) -> pipeline48/1(d)
  pipeline39/1(q) -> pipeline45/1(d)
  pipeline40/1(q) -> pipeline46/1(d)
  pipeline56/1(q) -> pfb_fft_1/5(pol3_in)
  pipeline55/1(q) -> pfb_fft_1/4(pol2_in)
  pipeline52/1(q) -> pfb_fft_1/2(pol0_in)
  pipeline54/1(q) -> pfb_fft_1/3(pol1_on)
  pipeline57/1(q) -> pfb_fft_1/1(sync)
  pipeline62/1(q) -> phase_1/1(sync)
  pipeline61/1(q) -> [fft_b1]
  pipeline60/1(q) -> [fft_b2]
  pipeline59/1(q) -> [fft_b3]
  pipeline53/1(q) -> [fft_b4]
  [fft_b1] -> phase_1/2(in1)
  [fft_b2] -> phase_1/3(in2)
  [fft_b3] -> phase_1/4(in3)
  [fft_b4] -> phase_1/5(in4)
  [adc16_chan_c] -> Bus Selector2/1
  Bus Selector2/1 -> pipeline84/1(d)  "<c1>"
  Bus Selector2/2 -> pipeline86/1(d)  "<c2>"
  Bus Selector2/3 -> pipeline87/1(d)  "<c3>"
  Bus Selector2/4 -> pipeline88/1(d)  "<c4>"
  [sync_gen] -> pipeline89/1(d)
  [cal_we] -> xpow2/1(we)
  [cal_addr] -> xpow2/2(addr)
  [cal_new_acc] -> xpow2/3(new_acc)
  xpow2/1(valid) -> Terminator12/1
  [sync_fft] -> pow_quant_2/1(sync)
  [c1] -> pow_quant_2/2(in0)
  [c2] -> pow_quant_2/3(in1)
  [c3] -> pow_quant_2/4(in2)
  [c4] -> pow_quant_2/5(in3)
  [gain] -> pow_quant_2/6(gain)
  pow_quant_2/1(sync_out) -> pipeline97/1(d)
  pow_quant_2/2(out0) -> pipeline96/1(d)
  pow_quant_2/3(out1) -> pipeline95/1(d)
  pow_quant_2/4(out2) -> pipeline69/1(d)
  pow_quant_2/5(out3) -> pipeline90/1(d)
  pipeline98/1(q) -> Terminator7/1
  [cal_we] -> xab2/1(we)
  [cal_addr] -> xab2/2(addr)
  [cal_new_acc] -> xab2/3(new_acc)
  xab2/1(valid) -> Terminator8/1
  [sync_fft] -> mul_quant_2/1(sync)
  [gain] -> mul_quant_2/7(gain)
  [a1] -> mul_quant_2/2(s_ref)
  [c1] -> mul_quant_2/3(s0)
  [c2] -> mul_quant_2/4(s1)
  [c3] -> mul_quant_2/5(s2)
  [c4] -> mul_quant_2/6(s3)
  mul_quant_2/1(sync_out) -> pipeline98/1(d)
  mul_quant_2/2(ab_re0) -> pipeline99/1(d)
  mul_quant_2/3(ab_im0) -> pipeline100/1(d)
  mul_quant_2/4(ab_re1) -> pipeline70/1(d)
  mul_quant_2/5(ab_im1) -> pipeline71/1(d)
  mul_quant_2/6(ab_re2) -> pipeline74/1(d)
  mul_quant_2/7(ab_im2) -> pipeline75/1(d)
  mul_quant_2/8(ab_re3) -> pipeline72/1(d)
  mul_quant_2/9(ab_im3) -> pipeline73/1(d)
  pfb_fft_2/1(sync_out) -> pipeline94/1(d)
  pfb_fft_2/2(pol0_out) -> pipeline93/1(d)
  pfb_fft_2/3(pol1_out) -> pipeline92/1(d)
  pfb_fft_2/4(pol2_out) -> pipeline91/1(d)
  pfb_fft_2/5(pol3_out) -> pipeline85/1(d)
  phase_2/1(sync_out) -> Terminator10/1
  phase_2/3(out2) -> [c2]
  phase_2/4(out3) -> [c3]
  phase_2/5(out4) -> [c4]
  phase_2/2(out1) -> [c1]
  [phase_cal_c1] -> phase_2/6(phase1)
  [phase_cal_c2] -> phase_2/7(phase2)
  [phase_cal_c3] -> phase_2/8(phase3)
  [phase_cal_c4] -> phase_2/9(phase4)
  pipeline97/1(q) -> Terminator9/1
  pipeline69/1(q) -> xpow2/6(din3)
  pipeline96/1(q) -> xpow2/4(din1)
  pipeline90/1(q) -> xpow2/7(din4)
  pipeline95/1(q) -> xpow2/5(din2)
  pipeline83/1(q) -> xab2/5(im0)
  pipeline78/1(q) -> xab2/10(re3)
  pipeline79/1(q) -> xab2/11(im3)
  pipeline80/1(q) -> xab2/8(re2)
  pipeline82/1(q) -> xab2/4(re0)
  pipeline81/1(q) -> xab2/9(im2)
  pipeline77/1(q) -> xab2/7(im1)
  pipeline76/1(q) -> xab2/6(re1)
  pipeline99/1(q) -> pipeline82/1(d)
  pipeline100/1(q) -> pipeline83/1(d)
  pipeline70/1(q) -> pipeline76/1(d)
  pipeline71/1(q) -> pipeline77/1(d)
  pipeline74/1(q) -> pipeline80/1(d)
  pipeline75/1(q) -> pipeline81/1(d)
  pipeline72/1(q) -> pipeline78/1(d)
  pipeline73/1(q) -> pipeline79/1(d)
  pipeline88/1(q) -> pfb_fft_2/5(pol3_in)
  pipeline87/1(q) -> pfb_fft_2/4(pol2_in)
  pipeline84/1(q) -> pfb_fft_2/2(pol0_in)
  pipeline86/1(q) -> pfb_fft_2/3(pol1_on)
  pipeline89/1(q) -> pfb_fft_2/1(sync)
  pipeline94/1(q) -> phase_2/1(sync)
  pipeline93/1(q) -> [fft_c1]
  pipeline92/1(q) -> [fft_c2]
  pipeline91/1(q) -> [fft_c3]
  pipeline85/1(q) -> [fft_c4]
  [fft_c1] -> phase_2/2(in1)
  [fft_c2] -> phase_2/3(in2)
  [fft_c3] -> phase_2/4(in3)
  [fft_c4] -> phase_2/5(in4)
  [adc16_chan_d] -> Bus Selector3/1
  Bus Selector3/1 -> pipeline117/1(d)  "<d1>"
  Bus Selector3/2 -> pipeline119/1(d)  "<d2>"
  Bus Selector3/3 -> pipeline120/1(d)  "<d3>"
  Bus Selector3/4 -> pipeline121/1(d)  "<d4>"
  [sync_gen] -> pipeline122/1(d)
  [cal_we] -> xpow3/1(we)
  [cal_addr] -> xpow3/2(addr)
  [cal_new_acc] -> xpow3/3(new_acc)
  xpow3/1(valid) -> Terminator14/1
  [sync_fft] -> pow_quant_3/1(sync)
  [d1] -> pow_quant_3/2(in0)
  [d2] -> pow_quant_3/3(in1)
  [d3] -> pow_quant_3/4(in2)
  [d4] -> pow_quant_3/5(in3)
  [gain] -> pow_quant_3/6(gain)
  pow_quant_3/1(sync_out) -> pipeline130/1(d)
  pow_quant_3/2(out0) -> pipeline129/1(d)
  pow_quant_3/3(out1) -> pipeline128/1(d)
  pow_quant_3/4(out2) -> pipeline102/1(d)
  pow_quant_3/5(out3) -> pipeline123/1(d)
  pipeline131/1(q) -> Terminator15/1
  [cal_we] -> xab3/1(we)
  [cal_addr] -> xab3/2(addr)
  [cal_new_acc] -> xab3/3(new_acc)
  xab3/1(valid) -> Terminator16/1
  [sync_fft] -> mul_quant_3/1(sync)
  [gain] -> mul_quant_3/7(gain)
  [a1] -> mul_quant_3/2(s_ref)
  [d1] -> mul_quant_3/3(s0)
  [d2] -> mul_quant_3/4(s1)
  [d3] -> mul_quant_3/5(s2)
  [d4] -> mul_quant_3/6(s3)
  mul_quant_3/1(sync_out) -> pipeline131/1(d)
  mul_quant_3/2(ab_re0) -> pipeline132/1(d)
  mul_quant_3/3(ab_im0) -> pipeline101/1(d)
  mul_quant_3/4(ab_re1) -> pipeline103/1(d)
  mul_quant_3/5(ab_im1) -> pipeline104/1(d)
  mul_quant_3/6(ab_re2) -> pipeline107/1(d)
  mul_quant_3/7(ab_im2) -> pipeline108/1(d)
  mul_quant_3/8(ab_re3) -> pipeline105/1(d)
  mul_quant_3/9(ab_im3) -> pipeline106/1(d)
  pfb_fft_3/1(sync_out) -> pipeline127/1(d)
  pfb_fft_3/2(pol0_out) -> pipeline126/1(d)
  pfb_fft_3/3(pol1_out) -> pipeline125/1(d)
  pfb_fft_3/4(pol2_out) -> pipeline124/1(d)
  pfb_fft_3/5(pol3_out) -> pipeline118/1(d)
  phase_3/1(sync_out) -> Terminator13/1
  phase_3/3(out2) -> [d2]
  phase_3/4(out3) -> [d3]
  phase_3/5(out4) -> [d4]
  phase_3/2(out1) -> [d1]
  [phase_cal_d1] -> phase_3/6(phase1)
  [phase_cal_d2] -> phase_3/7(phase2)
  [phase_cal_d3] -> phase_3/8(phase3)
  [phase_cal_d4] -> phase_3/9(phase4)
  pipeline130/1(q) -> Terminator17/1
  pipeline102/1(q) -> xpow3/6(din3)
  pipeline129/1(q) -> xpow3/4(din1)
  pipeline123/1(q) -> xpow3/7(din4)
  pipeline128/1(q) -> xpow3/5(din2)
  pipeline116/1(q) -> xab3/5(im0)
  pipeline111/1(q) -> xab3/10(re3)
  pipeline112/1(q) -> xab3/11(im3)
  pipeline113/1(q) -> xab3/8(re2)
  pipeline115/1(q) -> xab3/4(re0)
  pipeline114/1(q) -> xab3/9(im2)
  pipeline110/1(q) -> xab3/7(im1)
  pipeline109/1(q) -> xab3/6(re1)
  pipeline132/1(q) -> pipeline115/1(d)
  pipeline101/1(q) -> pipeline116/1(d)
  pipeline103/1(q) -> pipeline109/1(d)
  pipeline104/1(q) -> pipeline110/1(d)
  pipeline107/1(q) -> pipeline113/1(d)
  pipeline108/1(q) -> pipeline114/1(d)
  pipeline105/1(q) -> pipeline111/1(d)
  pipeline106/1(q) -> pipeline112/1(d)
  pipeline121/1(q) -> pfb_fft_3/5(pol3_in)
  pipeline120/1(q) -> pfb_fft_3/4(pol2_in)
  pipeline117/1(q) -> pfb_fft_3/2(pol0_in)
  pipeline119/1(q) -> pfb_fft_3/3(pol1_on)
  pipeline122/1(q) -> pfb_fft_3/1(sync)
  pipeline127/1(q) -> phase_3/1(sync)
  pipeline126/1(q) -> [fft_d1]
  pipeline125/1(q) -> [fft_d2]
  pipeline124/1(q) -> [fft_d3]
  pipeline118/1(q) -> [fft_d4]
  [fft_d1] -> phase_3/2(in1)
  [fft_d2] -> phase_3/3(in2)
  [fft_d3] -> phase_3/4(in3)
  [fft_d4] -> phase_3/5(in4)
